simulink model "HybPVWBFuzzy"  (R2017a)
settings: Solver=ode23tb  StartTime=0.0  StopTime=0.1  RelTol=1e-3  EnableMultiTasking=on
undefined symbols (not defined in the file): Acid, BMSS, c1, c2, c3, c4, c5, c6, Lead, pv10k, R, WM2
libraries: powerlib x61, powerlib_meascontrol x7, dspstat3 x4, fuzblock x3, powerlib_extras x3, simulink x2, electricdrivelib x1, re_lib x1
(part 1 of 2)
settings Model: Creator=Indhu  LastModifiedBy=venka
settings DataIOCC: LimitDataPoints=on  SaveFormat=Array
settings OptimizationCC: LifeSpan=inf
settings DebuggingCC: SolverPrmCheckMsg=warning  SFUnexpectedBacktrackingDiag=warning  SFNoUnconditionalDefaultTransitionDiag=warning  SFExecutionAtInitializationDiag=none
settings HardwareCC: ProdBitPerPointer=32  ProdBitPerSizeT=32  ProdBitPerPtrDiffT=32  ProdLargestAtomicFloat=None  ProdIntDivRoundTo=Undefined  ProdEndianess=Unspecified  ProdWordSize=32  ProdHWDeviceType=32-bit Generic
settings ModelReferenceCC: PropagateSignalLabelsOutOfModel=off
settings GRTTargetCC: TargetLangStandard=C89/C90 (ANSI)  GeneratePreprocessorConditionals=Disable all
settings ConfigComp: CovMetricSettings=dw  CovHtmlReporting=on  CovSaveCumulativeToWorkspaceVar=on  CovSaveSingleToWorkspaceVar=on  CovExternalEMLEnable=off  CovSFcnEnable=off

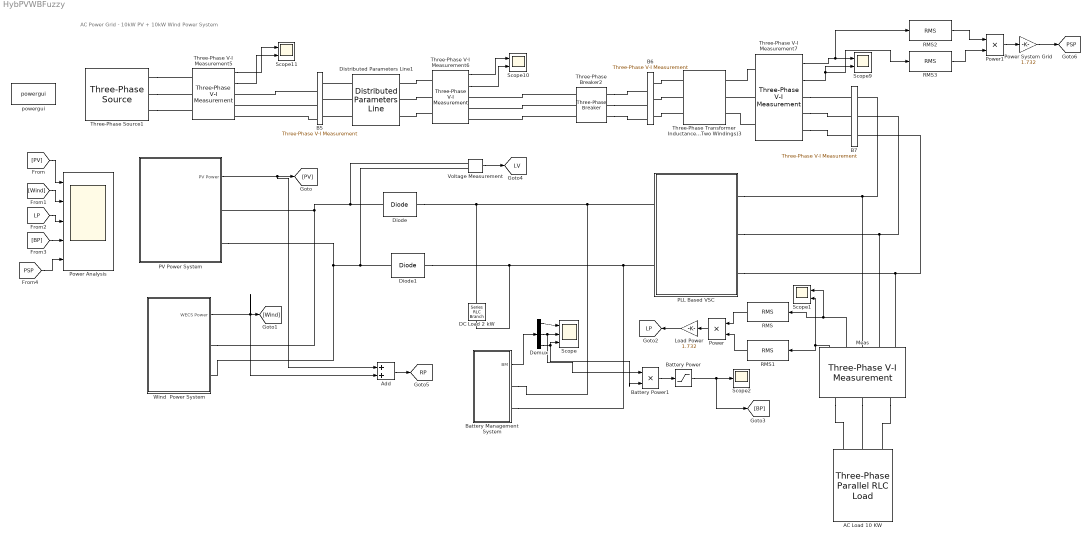
== system HybPVWBFuzzy ==
  note: AC Power Grid - 10kW PV + 10kW Wind Power System
  " Power" : Product
  " Power1" : Product
  AC Load 10 KW : powerlib/Elements/Three-Phase Parallel RLC Load  LConnTagsString=A|B|C  Configuration=Y (grounded)  NominalVoltage=200  Vabc=[1e3 1e3 1e3]  Vabcp=[1e3 1e3 1e3]  NominalFrequency=50  UnbalancedPower=off  ActivePower=30e3  InductivePower=10  CapacitivePower=0  Pabc=[10e3 9e3 11e3]  QLabc=[100 90 110]  QCabc=[100 90 110]  Pabcp=[10e3 9e3 11e3]  QLabcp=[100 90 110]  QCabcp=[100 90 110]  Measurements=None  LoadType=constant Z
  Add : Sum
  B5 : powerlib/Measurements/Three-Phase V-I Measurement  VoltageMeasurement=phase-to-ground  SetLabelV=off  LabelV=Vabc_B12  Vpu=off  VpuLL=off  CurrentMeasurement=no  SetLabelI=off  LabelI=A1  Ipu=off  Pbase=100e6  Vbase=25e3  OutputType=Magnitude  PhasorSimulation=off  PSBequivalent=0
  B6 : powerlib/Measurements/Three-Phase V-I Measurement  VoltageMeasurement=phase-to-phase  SetLabelV=off  LabelV=Vabc_B12  Vpu=off  VpuLL=off  CurrentMeasurement=no  SetLabelI=off  LabelI=A1  Ipu=off  Pbase=100e6  Vbase=440  OutputType=Magnitude  PhasorSimulation=off  PSBequivalent=0
  B7 : powerlib/Measurements/Three-Phase V-I Measurement  VoltageMeasurement=phase-to-ground  SetLabelV=on  LabelV=Vabc_B13  Vpu=off  VpuLL=off  CurrentMeasurement=yes  SetLabelI=on  LabelI=Iabc_b13  Ipu=off  Pbase=100e6  Vbase=440  OutputType=Magnitude  PhasorSimulation=off  PSBequivalent=0
  Battery Management System : Subsystem
  Battery Power : Saturate  UpperLimit=10000  LowerLimit=0
  Battery Power1 : Product
  DC Load 2 kW : powerlib/Elements/Series RLC Branch  LConnTagsString=__new0  RConnTagsString=__new0  BranchType=R  Resistance=2e3  Inductance=1.0  SetiL0=off  InitialCurrent=0  Capacitance=1.0  Setx0=off  InitialVoltage=0  Measurements=None
  Demux : Demux  Outputs=3
  Diode : powerlib/Power Electronics/Diode  Ron=0.001  Lon=0  Vf=0.8  IC=0  UseSnubber=0  Rs=500  Cs=250e-9  Measurements=off
  Diode1 : powerlib/Power Electronics/Diode  Ron=0.001  Lon=0  Vf=0.8  IC=0  UseSnubber=0  Rs=500  Cs=250e-9  Measurements=off
  Distributed Parameters Line1 : powerlib/Elements/Distributed Parameters Line  Phases=3  Frequency=50  Resistance=[0.01273 0.3864]  Inductance="[0.9337e-3  4.1264e-3]"  Capacitance=[12.74e-9 7.751e-9]  Length=100  x1=[-0.00056555 1.08831293548687e-09 21722.1182004137 -60866.0436678485;-0.00056554 1.08830572884738e-09 21722.3094166222 -60865.9754255012;-0.00056553 1.08829852219714e-09 21722.5006326162 -60865.9071825531;-0.00056552 1.08829131553617e-09 21722.6918483959 -60865.8389390044;-0.00056551 1.08828410886445e-09 21722.8830639612 -60865.7706948549;-0.0005655 1.08827690218199e-09 21723.074279312 -60865.7024501047;-0.00056549 1.08826969548879e-09 21723.2654944485 -60865.6342047537;-0.00056548 1.08826248878485e-09 21723.4567093706 -60865.5659588021;-0.00056547 1.08825528207017e-09 21723.6479240782 -60865.4977122497;-0.00056546 1.08824807534475e-09 21723.8391385715 -60865.4294650966;-0.00056545 1.08824086860859e-09 21724.0303528504 -60865.3612173428;-0.00056544 1.08823366186169e-09 21724.2215669148 -60865.2929689883;-0.00056543 1.08822645510405e-09 21724.4127807649 -60865.2247200331;-0.00056542 1.08821924833566e-09 21724.6039944005 -60865.1564704771;-0.00056541 1.08821204155654e-09 21724.7952078217 -60865.0882203205;-0.0005654 1.08820483476668e-09 21724.9864210285 -60865.0199695631;-0.00056539 1.08819762796607e-09 21725.1776340209 -60864.951718205;-0.00056538 1.08819042115473e-09 21725.3688467989 -60864.8834662462;-0.00056537 1.08818321433265e-09 21725.5600593624 -60864.8152136867;-0.00056536 1.08817600749982e-09 21725.7512717116 -60864.7469605264;-0.00056535 1.08816880065626e-09 21725.9424838463 -60864.6787067655;-0.00056534 1.08816159380196e-09 21726.1336957665 -60864.6104524038;-0.00056533 1.08815438693691e-09 21726.3249074724 -60864.5421974415;-0.00056532 1.08814718006113e-09 21726.5161189638 -60864.4739418784;-0.00056531 1.08813997317461e-09 21726.7073302408 -60864.4056857146;-0.0005653 1.08813276627735e-09 21726.8985413033 -60864.3374289501;-0.00056529 1.08812555936935e-09 21727.0897521514 -60864.2691715849;-0.00056528 1.0881183524506e-09 21727.2809627851 -60864.200913619;-0.00056527 1.08811114552112e-09 21727.4721732044 -60864.1326550524;-0.00056526 1.08810393858091e-09 21727.6633834092 -60864.0643958851;-0.00056525 1.08809673162995e-09 21727.8545933995 -60863.9961361171;-0.00056524 1.08808952466825e-09 21728.0458031754 -60863.9278757484;-0.00056523 1.08808231769581e-09 21728.2370127369 -60863.8596147789;-0.00056522 1.08807511071264e-09 21728.4282220839 -60863.7913532088;-0.00056521 1.08806790371873e-09 21728.6194312164 -60863.723091038;-0.0005652 1.08806069671407e-09 21728.8106401345 -60863.6548282665;-0.00056519 1.08805348969868e-09 21729.0018488382 -60863.5865648942;-0.00056518 1.08804628267255e-09 21729.1930573274 -60863.5183009213;-0.00056517 1.08803907563568e-09 21729.3842656021 -60863.4500363477;-0.00056516 1.08803186858807e-09 21729.5754736624 -60863.3817711734;-0.00056515 1.08802466152973e-09 21729.7666815082 -60863.3135053983;-0.00056514 1.08801745446064e-09 21729.9578891396 -60863.2452390226;-0.00056513 1.08801024738082e-09 21730.1490965565 -60863.1769720462;-0.00056512 1.08800304029026e-09 21730.3403037589 -60863.1087044691;-0.00056511 1.08799583318896e-09 21730.5315107468 -60863.0404362913;-0.0005651 1.08798862607693e-09 21730.7227175203 -60862.9721675128;-0.00056509 1.08798141895415e-09 21730.9139240793 -60862.9038981336;-0.00056508 1.08797421182064e-09 21731.1051304238 -60862.8356281537;-0.00056507 1.08796700467639e-09 21731.2963365539 -60862.7673575731;-0.00056506 1.0879597975214e-09 21731.4875424694 -60862.6990863918;-0.00056505 1.08795259035567e-09 21731.6787481705 -60862.6308146099;-0.00056504 1.08794538317921e-09 21731.8699536571 -60862.5625422272;-0.00056503 1.08793817599201e-09 21732.0611589292 -60862.4942692439;-0.00056502 1.08793096879407e-09 21732.2523639869 -60862.4259956599;-0.00056501 1.08792376158539e-09 21732.44356883 -60862.3577214751;-0.000565 1.08791655436598e-09 21732.6347734587 -60862.2894466897;-0.00056499 1.08790934713583e-09 21732.8259778728 -60862.2211713037;-0.00056498 1.08790213989494e-09 21733.0171820725 -60862.1528953169;-0.00056497 1.08789493264331e-09 21733.2083860577 -60862.0846187294 ...(+3805556 chars)]  x2=[-0.00056555 5.75329377740265e-09 21823.7633335048 -61290.3330055853;-0.00056554 5.75326459106246e-09 21823.955882657 -61290.2644439083;-0.00056553 5.75323540466548e-09 21824.1484315938 -61290.1958816264;-0.00056552 5.75320621821173e-09 21824.3409803152 -61290.1273187395;-0.00056551 5.75317703170119e-09 21824.5335288212 -61290.0587552478;-0.0005655 5.75314784513387e-09 21824.7260771119 -61289.9901911511;-0.00056549 5.75311865850977e-09 21824.9186251871 -61289.9216264496;-0.00056548 5.75308947182889e-09 21825.1111730469 -61289.8530611431;-0.00056547 5.75306028509123e-09 21825.3037206913 -61289.7844952317;-0.00056546 5.75303109829678e-09 21825.4962681203 -61289.7159287154;-0.00056545 5.75300191144556e-09 21825.6888153339 -61289.6473615943;-0.00056544 5.75297272453756e-09 21825.8813623321 -61289.5787938682;-0.00056543 5.75294353757278e-09 21826.0739091149 -61289.5102255372;-0.00056542 5.75291435055121e-09 21826.2664556822 -61289.4416566013;-0.00056541 5.75288516347287e-09 21826.4590020342 -61289.3730870605;-0.0005654 5.75285597633775e-09 21826.6515481707 -61289.3045169148;-0.00056539 5.75282678914586e-09 21826.8440940918 -61289.2359461641;-0.00056538 5.75279760189718e-09 21827.0366397975 -61289.1673748086;-0.00056537 5.75276841459173e-09 21827.2291852877 -61289.0988028482;-0.00056536 5.7527392272295e-09 21827.4217305626 -61289.0302302829;-0.00056535 5.75271003981049e-09 21827.614275622 -61288.9616571127;-0.00056534 5.7526808523347e-09 21827.806820466 -61288.8930833376;-0.00056533 5.75265166480214e-09 21827.9993650945 -61288.8245089576;-0.00056532 5.7526224772128e-09 21828.1919095076 -61288.7559339727;-0.00056531 5.75259328956669e-09 21828.3844537053 -61288.6873583829;-0.0005653 5.7525641018638e-09 21828.5769976875 -61288.6187821882;-0.00056529 5.75253491410413e-09 21828.7695414543 -61288.5502053887;-0.00056528 5.75250572628769e-09 21828.9620850057 -61288.4816279842;-0.00056527 5.75247653841448e-09 21829.1546283416 -61288.4130499748;-0.00056526 5.75244735048449e-09 21829.347171462 -61288.3444713606;-0.00056525 5.75241816249772e-09 21829.539714367 -61288.2758921414;-0.00056524 5.75238897445418e-09 21829.7322570566 -61288.2073123174;-0.00056523 5.75235978635387e-09 21829.9247995307 -61288.1387318884;-0.00056522 5.75233059819678e-09 21830.1173417894 -61288.0701508546;-0.00056521 5.75230140998292e-09 21830.3098838326 -61288.0015692159;-0.0005652 5.75227222171229e-09 21830.5024256604 -61287.9329869723;-0.00056519 5.75224303338488e-09 21830.6949672727 -61287.8644041238;-0.00056518 5.75221384500071e-09 21830.8875086695 -61287.7958206704;-0.00056517 5.75218465655976e-09 21831.0800498509 -61287.7272366122;-0.00056516 5.75215546806204e-09 21831.2725908168 -61287.658651949;-0.00056515 5.75212627950754e-09 21831.4651315672 -61287.590066681;-0.00056514 5.75209709089628e-09 21831.6576721022 -61287.5214808081;-0.00056513 5.75206790222824e-09 21831.8502124217 -61287.4528943303;-0.00056512 5.75203871350344e-09 21832.0427525257 -61287.3843072476;-0.00056511 5.75200952472186e-09 21832.2352924143 -61287.31571956;-0.0005651 5.75198033588351e-09 21832.4278320874 -61287.2471312676;-0.00056509 5.7519511469884e-09 21832.620371545 -61287.1785423702;-0.00056508 5.75192195803651e-09 21832.8129107871 -61287.109952868;-0.00056507 5.75189276902786e-09 21833.0054498138 -61287.041362761;-0.00056506 5.75186357996244e-09 21833.1979886249 -61286.972772049;-0.00056505 5.75183439084024e-09 21833.3905272206 -61286.9041807321;-0.00056504 5.75180520166128e-09 21833.5830656008 -61286.8355888104;-0.00056503 5.75177601242555e-09 21833.7756037655 -61286.7669962838;-0.00056502 5.75174682313306e-09 21833.9681417147 -61286.6984031523;-0.00056501 5.75171763378379e-09 21834.1606794484 -61286.629809416;-0.000565 5.75168844437776e-09 21834.3532169667 -61286.5612150748;-0.00056499 5.75165925491497e-09 21834.5457542694 -61286.4926201287;-0.00056498 5.7516300653954e-09 21834.7382913566 -61286.4240245777;-0.00056497 5.75160087581907e-09 21834.9308282283 -61286.3554284219;-0.00056496 ...(+3805385 chars)]  x3=[-0.00056555 5.97680860293045e-11 53.5100222471423 -232.462000464429;-0.00056554 5.97681336253323e-11 53.5107525477912 -232.461832356589;-0.00056553 5.97681812207703e-11 53.5114828479119 -232.461664246455;-0.00056552 5.97682288156184e-11 53.5122131475044 -232.461496134026;-0.00056551 5.97682764098766e-11 53.5129434465689 -232.461328019304;-0.0005655 5.97683240035449e-11 53.5136737451051 -232.461159902286;-0.00056549 5.97683715966233e-11 53.5144040431133 -232.460991782975;-0.00056548 5.97684191891118e-11 53.5151343405932 -232.460823661369;-0.00056547 5.97684667810105e-11 53.515864637545 -232.460655537469;-0.00056546 5.97685143723192e-11 53.5165949339686 -232.460487411275;-0.00056545 5.97685619630381e-11 53.517325229864 -232.460319282786;-0.00056544 5.9768609553167e-11 53.5180555252312 -232.460151152003;-0.00056543 5.97686571427061e-11 53.5187858200702 -232.459983018926;-0.00056542 5.97687047316553e-11 53.519516114381 -232.459814883555;-0.00056541 5.97687523200146e-11 53.5202464081636 -232.459646745889;-0.0005654 5.9768799907784e-11 53.5209767014179 -232.459478605929;-0.00056539 5.97688474949635e-11 53.5217069941441 -232.459310463674;-0.00056538 5.9768895081553e-11 53.522437286342 -232.459142319126;-0.00056537 5.97689426675528e-11 53.5231675780116 -232.458974172283;-0.00056536 5.97689902529626e-11 53.523897869153 -232.458806023146;-0.00056535 5.97690378377825e-11 53.5246281597661 -232.458637871714;-0.00056534 5.97690854220125e-11 53.525358449851 -232.458469717988;-0.00056533 5.97691330056526e-11 53.5260887394076 -232.458301561968;-0.00056532 5.97691805887028e-11 53.5268190284359 -232.458133403654;-0.00056531 5.97692281711631e-11 53.5275493169359 -232.457965243045;-0.0005653 5.97692757530336e-11 53.5282796049077 -232.457797080143;-0.00056529 5.97693233343141e-11 53.5290098923511 -232.457628914946;-0.00056528 5.97693709150047e-11 53.5297401792662 -232.457460747454;-0.00056527 5.97694184951054e-11 53.530470465653 -232.457292577669;-0.00056526 5.97694660746163e-11 53.5312007515115 -232.457124405589;-0.00056525 5.97695136535372e-11 53.5319310368416 -232.456956231214;-0.00056524 5.97695612318682e-11 53.5326613216434 -232.456788054546;-0.00056523 5.97696088096093e-11 53.5333916059169 -232.456619875583;-0.00056522 5.97696563867605e-11 53.534121889662 -232.456451694327;-0.00056521 5.97697039633219e-11 53.5348521728787 -232.456283510775;-0.0005652 5.97697515392933e-11 53.5355824555671 -232.45611532493;-0.00056519 5.97697991146748e-11 53.5363127377271 -232.45594713679;-0.00056518 5.97698466894664e-11 53.5370430193587 -232.455778946356;-0.00056517 5.97698942636681e-11 53.5377733004619 -232.455610753628;-0.00056516 5.97699418372799e-11 53.5385035810368 -232.455442558606;-0.00056515 5.97699894103018e-11 53.5392338610832 -232.455274361289;-0.00056514 5.97700369827337e-11 53.5399641406012 -232.455106161678;-0.00056513 5.97700845545758e-11 53.5406944195908 -232.454937959773;-0.00056512 5.9770132125828e-11 53.5414246980519 -232.454769755574;-0.00056511 5.97701796964902e-11 53.5421549759847 -232.45460154908;-0.0005651 5.97702272665626e-11 53.542885253389 -232.454433340292;-0.00056509 5.9770274836045e-11 53.5436155302648 -232.45426512921;-0.00056508 5.97703224049376e-11 53.5443458066122 -232.454096915834;-0.00056507 5.97703699732402e-11 53.5450760824312 -232.453928700163;-0.00056506 5.97704175409529e-11 53.5458063577216 -232.453760482199;-0.00056505 5.97704651080757e-11 53.5465366324836 -232.45359226194;-0.00056504 5.97705126746086e-11 53.5472669067171 -232.453424039386;-0.00056503 5.97705602405516e-11 53.5479971804222 -232.453255814539;-0.00056502 5.97706078059047e-11 53.5487274535987 -232.453087587397;-0.00056501 5.97706553706678e-11 53.5494577262467 -232.452919357961;-0.000565 5.97707029348411e-11 53.5501879983662 -232.452751126231;-0.00056499 5.97707504984244e-11 53.5509182699572 -232.452582892207;-0.00056498 5.97707980614178e-11 53.5516485410196 -232.452414655888;-0.00056497 5.97708456238213e-11 53.5523788115536 -232.452246417276;-0.00056496 ...(+3805462 chars)]  x4=[-0.00056555 -4.95982915055118e-11 82.3635169225828 -225.879807325379;-0.00056554 -4.95983839978684e-11 82.3642265445196 -225.879548571644;-0.00056553 -4.95984764897355e-11 82.3649361656436 -225.879289815681;-0.00056552 -4.9598568981113e-11 82.3656457859546 -225.879031057488;-0.00056551 -4.9598661472001e-11 82.3663554054527 -225.878772297065;-0.0005655 -4.95987539623995e-11 82.3670650241379 -225.878513534413;-0.00056549 -4.95988464523085e-11 82.3677746420101 -225.878254769532;-0.00056548 -4.95989389417279e-11 82.3684842590695 -225.877996002422;-0.00056547 -4.95990314306579e-11 82.3691938753158 -225.877737233082;-0.00056546 -4.95991239190983e-11 82.3699034907493 -225.877478461513;-0.00056545 -4.95992164070492e-11 82.3706131053697 -225.877219687715;-0.00056544 -4.95993088945105e-11 82.3713227191772 -225.876960911687;-0.00056543 -4.95994013814824e-11 82.3720323321717 -225.87670213343;-0.00056542 -4.95994938679647e-11 82.3727419443533 -225.876443352944;-0.00056541 -4.95995863539574e-11 82.3734515557219 -225.876184570228;-0.0005654 -4.95996788394607e-11 82.3741611662774 -225.875925785284;-0.00056539 -4.95997713244744e-11 82.37487077602 -225.875666998109;-0.00056538 -4.95998638089986e-11 82.3755803849496 -225.875408208706;-0.00056537 -4.95999562930333e-11 82.3762899930661 -225.875149417073;-0.00056536 -4.96000487765784e-11 82.3769996003696 -225.874890623211;-0.00056535 -4.9600141259634e-11 82.3777092068601 -225.874631827119;-0.00056534 -4.96002337422001e-11 82.3784188125376 -225.874373028799;-0.00056533 -4.96003262242766e-11 82.379128417402 -225.874114228249;-0.00056532 -4.96004187058636e-11 82.3798380214534 -225.873855425469;-0.00056531 -4.96005111869611e-11 82.3805476246917 -225.873596620461;-0.0005653 -4.9600603667569e-11 82.3812572271169 -225.873337813223;-0.00056529 -4.96006961476874e-11 82.3819668287291 -225.873079003756;-0.00056528 -4.96007886273162e-11 82.3826764295282 -225.872820192059;-0.00056527 -4.96008811064555e-11 82.3833860295142 -225.872561378134;-0.00056526 -4.96009735851053e-11 82.3840956286872 -225.872302561979;-0.00056525 -4.96010660632655e-11 82.384805227047 -225.872043743595;-0.00056524 -4.96011585409362e-11 82.3855148245937 -225.871784922981;-0.00056523 -4.96012510181173e-11 82.3862244213273 -225.871526100138;-0.00056522 -4.96013434948089e-11 82.3869340172478 -225.871267275066;-0.00056521 -4.96014359710109e-11 82.3876436123552 -225.871008447765;-0.0005652 -4.96015284467234e-11 82.3883532066494 -225.870749618234;-0.00056519 -4.96016209219464e-11 82.3890628001305 -225.870490786475;-0.00056518 -4.96017133966798e-11 82.3897723927984 -225.870231952486;-0.00056517 -4.96018058709236e-11 82.3904819846532 -225.869973116267;-0.00056516 -4.96018983446779e-11 82.3911915756949 -225.86971427782;-0.00056515 -4.96019908179427e-11 82.3919011659233 -225.869455437143;-0.00056514 -4.96020832907179e-11 82.3926107553386 -225.869196594237;-0.00056513 -4.96021757630035e-11 82.3933203439407 -225.868937749102;-0.00056512 -4.96022682347996e-11 82.3940299317296 -225.868678901737;-0.00056511 -4.96023607061062e-11 82.3947395187053 -225.868420052143;-0.0005651 -4.96024531769231e-11 82.3954491048678 -225.86816120032;-0.00056509 -4.96025456472506e-11 82.3961586902171 -225.867902346268;-0.00056508 -4.96026381170884e-11 82.3968682747532 -225.867643489987;-0.00056507 -4.96027305864367e-11 82.3975778584761 -225.867384631476;-0.00056506 -4.96028230552955e-11 82.3982874413857 -225.867125770736;-0.00056505 -4.96029155236647e-11 82.3989970234821 -225.866866907767;-0.00056504 -4.96030079915443e-11 82.3997066047652 -225.866608042569;-0.00056503 -4.96031004589344e-11 82.4004161852351 -225.866349175141;-0.00056502 -4.96031929258349e-11 82.4011257648918 -225.866090305484;-0.00056501 -4.96032853922458e-11 82.4018353437351 -225.865831433598;-0.000565 -4.96033778581672e-11 82.4025449217652 -225.865572559483;-0.00056499 -4.9603470323599e-11 82.403254498982 -225.865313683139;-0.00056498 -4.96035627885412e-11 82.4039640753855 -225.865054804565;-0.00056497 ...(+3862159 chars)]  x5=0.000565541566995743  V1=[0 0 0 2.607e-09 6.463e+04 6.463e+04;0 0 0 314.2 314.2 314.2;1.571 1.571 1.571 -1.068 0.4127 -1.158]  V2=[0 0 0 1.109e-08 6.507e+04 6.507e+04;0 0 0 314.2 314.2 314.2;1.571 1.571 1.571 -1.193 0.4123 -1.158]  I1=[0 0 0 6.24e-11 239.5 239.5;0 0 0 314.2 314.2 314.2;1.571 1.571 1.571 -2.459 0.2963 -1.275]  I2=[0 0 0 5.82e-11 239.6 239.6;0 0 0 314.2 314.2 314.2;1.571 1.571 1.571 0.3876 0.4202 -1.151]  nHarmo=2  Measurements=None
      settings: SourceProductName=Simscape Power Systems
  Load Power : Gain  Gain=1.732
  Meas : powerlib/Measurements/Three-Phase V-I Measurement  VoltageMeasurement=phase-to-ground  SetLabelV=off  LabelV=Vabc_b1  Vpu=off  VpuLL=off  CurrentMeasurement=yes  SetLabelI=off  LabelI=Iabc_b1  Ipu=off  Pbase=100e6  Vbase=500e3  OutputType=Complex  PhasorSimulation=off  PSBequivalent=0
  "PLL Based VSC " : Subsystem
  "PV Power System " : Subsystem
  Power Analysis : Scope  NumInputPorts=5
  "Power System Grid " : Gain  Gain=1.732
  RMS{,1..3} (4 alike) : dspstat3/RMS  run=off  directionMode=Each column  dimension=1  InputProcessing=Columns as channels (frame based)  reset_popup=None
  Scope : Scope  NumInputPorts=3
  Scope{1,9..11} (4 alike) : Scope  NumInputPorts=2
  Scope2 : Scope
  Three-Phase V-I Measurement5 : powerlib/Measurements/Three-Phase V-I Measurement  VoltageMeasurement=phase-to-ground  SetLabelV=off  LabelV=Vabc  Vpu=off  VpuLL=off  CurrentMeasurement=yes  SetLabelI=off  LabelI=Iabc  Ipu=off  Pbase=100e6  Vbase=500e3  OutputType=Complex  PhasorSimulation=off  PSBequivalent=0
  Three-Phase V-I Measurement6 : powerlib/Measurements/Three-Phase V-I Measurement  VoltageMeasurement=phase-to-ground  SetLabelV=off  LabelV=Vabc  Vpu=off  VpuLL=off  CurrentMeasurement=yes  SetLabelI=off  LabelI=Iabc  Ipu=off  Pbase=100e6  Vbase=500e3  OutputType=Complex  PhasorSimulation=off  PSBequivalent=0
  Three-Phase V-I Measurement7 : powerlib/Measurements/Three-Phase V-I Measurement  VoltageMeasurement=phase-to-ground  SetLabelV=off  LabelV=Vabc_b1  Vpu=off  VpuLL=off  CurrentMeasurement=yes  SetLabelI=off  LabelI=Iabc_b1  Ipu=off  Pbase=100e6  Vbase=500e3  OutputType=Complex  PhasorSimulation=off  PSBequivalent=0
  Three-Phase Breaker2 : powerlib/Elements/Three-Phase Breaker  InitialState=closed  SwitchA=off  SwitchB=off  SwitchC=off  SwitchTimes="[1/50  20/50]"  External=off  BreakerResistance=0.001  SnubberResistance=1e6  SnubberCapacitance=inf  Measurements=None
  Three-Phase Source1 : powerlib/Electrical Sources/Three-Phase Source  InternalConnection=Yg  VoltagePhases=off  Voltage=63510.4  PhaseAngle=0  Voltage_phases=[25e3 25e3 25e3]/sqrt(3)  PhaseAngles_phases=[0 -120 +120]  Frequency=50  NonIdealSource=on  SpecifyImpedance=on  Resistance=0.8929  Inductance=16.58e-3  ShortCircuitLevel=100e6  BaseVoltage=63510.4  XRratio=7  BusType=swing  Pref=0  Qref=0  Prefabc=[10e3, 10e3, 10e3]  Qrefabc=[0, 0, 0]  Qmin=-inf  Qmax=inf
  Three-Phase Transformer Inductance Matrix Type (Two Windings)3 : powerlib/Elements/Three-Phase Transformer Inductance Matrix Type (Two Windings)  CoreType=Three-limb or five-limb core  Winding1Connection=Yg  Winding2Connection=Yg  AutoTransformer=off  Measurements=None  NominalPower=[100e3 50]  VLLnom=[63510.4 254.03]  WindingResistances=[0.01 0.01]  NoLoadIexcPos=2  NoLoadPlossPos=1000  ShortCircuitReactancePos=0.06  ShortCircuitReactancePosAuto=0.06  NoLoadIexcZero=100  NoLoadPlossZero=1500  ShortCircuitReactanceZero=0.03  ShortCircuitReactanceZeroAuto=0.03
      settings: SourceProductName=Simscape Power Systems  SourceProductBaseCode=PS
  Voltage Measurement : powerlib/Measurements/Voltage Measurement  PhasorSimulation=off  OutputType=Complex  PSBequivalent=0
  "Wind  Power System " : Subsystem
  powergui : powerlib/powergui  Priority=1  SimulationMode=Discrete  SampleTime=1e-8  frequency=60  Iterations=50  frequencyindice=50  Pbase=100e6  ErrMax=1e-4  UnitsV=kV  UnitsW=MW  buscounter=0  FunctionMessages=off  echomessages=off  EnableUseOfTLC=off  CurrentSourceSwitches=off  DisableSnubberDevices=off  DisableRonSwitches=off  DisableVfSwitches=off  DisplayEquations=off  SolverType=Tustin/Backward Euler (TBE)  Interpol=off  ExternalGateDelay=off  methode=off  Ts=100  x0status=blocks  SPID=on  SwTol=0  HookPort=off  RestoreLinks=warning  ResistiveCurrentMeasurement=off  Frange=[0:2:500]  Ylog=off  Xlog=on  ShowGrid=off  save=off  variable=ZData  structure=LVC  ZoomFFT=off  StartTime=0.02  cycles=2  DisplayStyle=1  fundamental=50  FreqAxis=off  MaxFrequency=1000  frequencyindicesteady=1  RmsSteady=1  display=off
  tag scope: LP=global, LV=global, PSP=global, RP=global
  wires:
  Three-Phase V-I Measurement6/1 -> Scope10/1
  Three-Phase V-I Measurement6/2 -> Scope10/2
  Three-Phase V-I Measurement5/1 -> Scope11/1
  Three-Phase V-I Measurement5/2 -> Scope11/2
  Three-Phase V-I Measurement7/1 -> RMS2/1, Scope9/1
  Battery Management System/1("BM ") -> Demux/1
  Meas/2 -> RMS/1, Scope1/1
  Meas/1 -> RMS1/1, Scope1/2
  RMS/1 -> " Power"/1
  RMS1/1 -> " Power"/2
  "PV Power System "/1(PV Power) -> Add/1, [PV]
  "Wind  Power System "/1(WECS Power) -> Add/2, [Wind]
  [PV] -> Power Analysis/1
  [Wind] -> Power Analysis/2
  [LP] -> Power Analysis/3
  [BP] -> Power Analysis/4
  Demux/1 -> Scope/1
  Demux/2 -> Battery Power1/1, Scope/2
  Demux/3 -> Battery Power1/2, Scope/3
  Battery Power1/1 -> Battery Power/1
  Battery Power/1 -> [BP], Scope2/1
  " Power"/1 -> Load Power/1
  Load Power/1 -> [LP]
  Voltage Measurement/1 -> [LV]
  Three-Phase V-I Measurement7/2 -> RMS3/1, Scope9/2
  RMS2/1 -> " Power1"/1
  RMS3/1 -> " Power1"/2
  " Power1"/1 -> "Power System Grid "/1
  "Power System Grid "/1 -> [PSP]
  [PSP] -> Power Analysis/5
  Add/1 -> [RP]
  connections:
  Three-Phase Breaker2/LConn3 -- B6/RConn3
  Three-Phase V-I Measurement6/LConn1 -- Distributed Parameters Line1/RConn1
  Three-Phase V-I Measurement7/RConn3 -- B7/RConn3
  Three-Phase Transformer Inductance Matrix Type (Two Windings)3/LConn1 -- B6/LConn1
  B5/RConn1 -- Three-Phase V-I Measurement5/RConn1
  Three-Phase Source1/RConn3 -- Three-Phase V-I Measurement5/LConn3
  Three-Phase Breaker2/RConn1 -- Three-Phase V-I Measurement6/RConn1
  Three-Phase Source1/RConn1 -- Three-Phase V-I Measurement5/LConn1
  Three-Phase V-I Measurement6/LConn2 -- Distributed Parameters Line1/RConn2
  Three-Phase Transformer Inductance Matrix Type (Two Windings)3/LConn3 -- B6/LConn3
  Three-Phase Breaker2/LConn1 -- B6/RConn1
  Three-Phase Breaker2/LConn2 -- B6/RConn2
  Three-Phase Breaker2/RConn2 -- Three-Phase V-I Measurement6/RConn2
  Three-Phase V-I Measurement6/LConn3 -- Distributed Parameters Line1/RConn3
  Three-Phase Transformer Inductance Matrix Type (Two Windings)3/LConn2 -- B6/LConn2
  Distributed Parameters Line1/LConn1 -- B5/LConn1
  B5/RConn2 -- Three-Phase V-I Measurement5/RConn2
  Three-Phase V-I Measurement7/LConn2 -- Three-Phase Transformer Inductance Matrix Type (Two Windings)3/RConn2
  Three-Phase V-I Measurement7/LConn3 -- Three-Phase Transformer Inductance Matrix Type (Two Windings)3/RConn3
  Distributed Parameters Line1/LConn2 -- B5/LConn2
  Distributed Parameters Line1/LConn3 -- B5/LConn3
  B5/RConn3 -- Three-Phase V-I Measurement5/RConn3
  Three-Phase V-I Measurement7/RConn2 -- B7/RConn2
  Three-Phase Breaker2/RConn3 -- Three-Phase V-I Measurement6/RConn3
  Three-Phase V-I Measurement7/LConn1 -- Three-Phase Transformer Inductance Matrix Type (Two Windings)3/RConn1
  Three-Phase V-I Measurement7/RConn1 -- B7/RConn1
  Three-Phase Source1/RConn2 -- Three-Phase V-I Measurement5/LConn2
  "PLL Based VSC "/RConn1 -- Meas/RConn1 -- B7/LConn1
  "PLL Based VSC "/RConn2 -- Meas/RConn2 -- B7/LConn2
  "PLL Based VSC "/RConn3 -- Meas/RConn3 -- B7/LConn3
  DC Load 2 kW/LConn1 -- Diode/RConn1 -- Battery Management System/RConn1 -- "PLL Based VSC "/LConn1
  DC Load 2 kW/RConn1 -- Diode1/RConn1 -- Battery Management System/RConn2 -- "PLL Based VSC "/LConn2
  Meas/LConn1 -- AC Load 10 KW/LConn1
  Meas/LConn2 -- AC Load 10 KW/LConn2
  Meas/LConn3 -- AC Load 10 KW/LConn3
  "Wind  Power System "/RConn1 -- Voltage Measurement/LConn1 -- Diode/LConn1 -- "PV Power System "/RConn1
  "PV Power System "/RConn2 -- Voltage Measurement/LConn2 -- Diode1/LConn1 -- "Wind  Power System "/RConn2
